simulink model "wlan_phy_rx_pmd"  (Simulink 7.8) (continued)
== system wlan_phy_rx_pmd/FCS & Pkt Buf/Pkt Buf Interface ==
  DSSS data : Inport
  OFDM data : Inport  Port=2
  BRAM IF 64b : Subsystem
  Bus Selector : BusSelector  OutputSignals=byte_valid,byte_index,byte,last_byte
  Bus Selector1 : BusSelector  OutputSignals=byte_valid,byte_index,byte,last_byte
  DSSS Addr Calc : Subsystem
  Logical7 : xbsIndex_r4/Logical  logical_function=OR  inputs=2  en=off  latency=0  precision=Full  arith_type=Unsigned  n_bits=8  bin_pt=2  align_bp=on  dbl_ovrd=off  block_type=logical
      settings: xl_area=[0, 0, 0, 0, 0, 0, 0]  block_version=VER_STRING_GOES_HERE
  MPDU Byte Outputs : Subsystem
  Mux2 : xbsIndex_r4/Mux  inputs=2  en=off  latency=0  precision=Full  arith_type=Unsigned  n_bits=16  bin_pt=14  quantization=Truncate  overflow=Wrap  dbl_ovrd=off  block_type=mux
  Mux3 : xbsIndex_r4/Mux  inputs=2  en=off  latency=0  precision=Full  arith_type=Unsigned  n_bits=16  bin_pt=14  quantization=Truncate  overflow=Wrap  dbl_ovrd=off  block_type=mux
  Mux5 : xbsIndex_r4/Mux  inputs=2  en=off  latency=0  precision=Full  arith_type=Unsigned  n_bits=16  bin_pt=14  quantization=Truncate  overflow=Wrap  dbl_ovrd=off  block_type=mux
  OFDM Addr Calc : Subsystem
  Register{4..5,7..8} (4 alike) : xbsIndex_r4/Register  init=0  rst=off  en=off  dbl_ovrd=off  block_type=register
  wires:
  [DSSS_Byte_Valid] -> Mux3/1
  [DSSS_Byte_Index] -> DSSS Addr Calc/1(R)
  DSSS Addr Calc/1(M) -> Mux5/3
  [DSSS_Byte_Valid] -> Mux5/1
  Mux5/1 -> Register5/1
  [OFDM_Byte_Index] -> OFDM Addr Calc/1(R)
  OFDM Addr Calc/1(M) -> Mux5/2
  Mux3/1 -> Register4/1
  Register4/1 -> BRAM IF 64b/4(Pkt Buf Sel)
  [CUR_RX_BYTE] -> Register7/1
  [CUR_RX_BYTE_VALID] -> Register8/1
  Register7/1 -> BRAM IF 64b/1("byte "), MPDU Byte Outputs/1("Byte ")
  Register8/1 -> BRAM IF 64b/2(byte_valid), MPDU Byte Outputs/2(Byte Valid)
  Register5/1 -> BRAM IF 64b/3(byte_ind), MPDU Byte Outputs/3(Byte Ind)
  Bus Selector1/1 -> [DSSS_Byte_Valid]  "<byte_valid>"
  Bus Selector1/2 -> [DSSS_Byte_Index]  "<byte_index>"
  Bus Selector1/3 -> [DSSS_Byte]  "<byte>"
  Bus Selector1/4 -> [DSSS_Last_Byte]  "<last_byte>"
  Bus Selector/1 -> [OFDM_Byte_Valid]  "<byte_valid>"
  Bus Selector/2 -> [OFDM_Byte_Index]  "<byte_index>"
  Bus Selector/3 -> [OFDM_Byte]  "<byte>"
  Bus Selector/4 -> [OFDM_Last_Byte]  "<last_byte>"
  DSSS data/1 -> Bus Selector1/1
  OFDM data/1 -> Bus Selector/1
  [DSSS_Byte_Valid] -> Mux2/1
  [OFDM_Byte] -> Mux2/2
  [DSSS_Byte] -> Mux2/3
  Mux2/1 -> [CUR_RX_BYTE]
  [DSSS_Byte_Valid] -> Logical7/1
  [OFDM_Byte_Valid] -> Logical7/2
  Logical7/1 -> [CUR_RX_BYTE_VALID]
  [regRx_OFDM_PKT_BUF_SEL] -> Mux3/2
  [regRx_DSSS_PKT_BUF_SEL] -> Mux3/3

== system wlan_phy_rx_pmd/FFT/Reset Gen ==
  note: Extend FFT reset for duration of TVALID + 4 to handle
      | case of pkt ending while new FFT frame is being loaded.
      | Turns out reset inputs for FIFO and FFT do not actually
      | flush internal state - both cores keep respnding to inputs
      | while reset is asserted (very frustrating...)
  FFT Valid In : Inport
  Reset : Inport  Port=2
  Inverter : xbsIndex_r4/Inverter  en=off  latency=0  dbl_ovrd=off  block_type=inv
  Inverter1 : xbsIndex_r4/Inverter  en=off  latency=4  dbl_ovrd=off  block_type=inv
  Logical : xbsIndex_r4/Logical  logical_function=AND  inputs=2  en=off  latency=0  precision=Full  arith_type=Unsigned  n_bits=16  bin_pt=0  align_bp=on  dbl_ovrd=off  block_type=logical
  Logical1 : xbsIndex_r4/Logical  logical_function=OR  inputs=2  en=off  latency=0  precision=Full  arith_type=Unsigned  n_bits=16  bin_pt=0  align_bp=on  dbl_ovrd=off  block_type=logical
  Register2 : xbsIndex_r4/Register  init=1  rst=off  en=off  dbl_ovrd=off  block_type=register
      settings: xl_area=[1 1 0 0 0 0 0]  block_version=VER_STRING_GOES_HERE
  S-R Latch1 : Subsystem
  FFT Reset : Outport
  " Reset" : Outport  Port=2
  wires:
  Inverter1/1 -> S-R Latch1/2(R)
  Reset/1 -> Logical1/1, Logical/2
  Logical/1 -> S-R Latch1/1(S)
  S-R Latch1/1(Q) -> Logical1/2
  Logical1/1 -> " Reset"/1, Inverter/1
  FFT Valid In/1 -> Inverter1/1, Logical/1
  Inverter/1 -> Register2/1
  Register2/1 -> FFT Reset/1

== system wlan_phy_rx_pmd/FFT/Sym Count ==
  data_in_tvalid : Inport
  pkt_done : Inport  Port=2
  data_out_tlast : Inport  Port=3
  Logical : xbsIndex_r4/Logical  logical_function=AND  inputs=2  en=off  latency=0  precision=Full  arith_type=Unsigned  n_bits=16  bin_pt=0  align_bp=on  dbl_ovrd=off  block_type=logical
  S-R Latch1 : Subsystem
  Symbol Counter : xbsIndex_r4/Counter  cnt_type=Free Running  cnt_to=Inf  operation=Up  start_count=0  cnt_by_val=1  arith_type=Unsigned  n_bits=ceil(log2(MAX_NUM_SYMS))  bin_pt=0  load_pin=off  rst=on  en=on  explicit_period=off  period=1  dbl_ovrd=off  use_behavioral_HDL=off  implementation=Fabric  block_type=counter
  Sym Ind : Outport
  wires:
  data_out_tlast/1 -> Logical/1
  Logical/1 -> Symbol Counter/2
  S-R Latch1/1(Q) -> Logical/2
  data_in_tvalid/1 -> S-R Latch1/1(S)
  Symbol Counter/1 -> Sym Ind/1
  pkt_done/1 -> S-R Latch1/2(R), Symbol Counter/1

== system wlan_phy_rx_pmd/Inputs/RF A RSSI Sum ==
  RSSI : Inport
  Down Sample6 : xbsIndex_r4/Down Sample  sample_ratio=16  sample_phase="Last Value of Frame  (most efficient)"  en=off  latency=1  dbl_ovrd=off  block_type=dsamp
      settings: block_version=VER_STRING_GOES_HERE
  RSSI DET Debug Out : Subsystem
  Running Sum : Subsystem
  Sum : Outport
  wires:
  [RX_GLOBAL_RESET] -> Down Sample6/1
  RSSI/1 -> Running Sum/1(RSSI), RSSI DET Debug Out/2(Raw RSSI)
  Down Sample6/1 -> Running Sum/2(reset)
  Running Sum/1(avg) -> Sum/1, RSSI DET Debug Out/1(Avg RSSI)

== system wlan_phy_rx_pmd/Inputs/RF B RSSI Sum ==
  RSSI : Inport
  Down Sample6 : xbsIndex_r4/Down Sample  sample_ratio=16  sample_phase="Last Value of Frame  (most efficient)"  en=off  latency=1  dbl_ovrd=off  block_type=dsamp
      settings: block_version=VER_STRING_GOES_HERE
  Running Sum : Subsystem
  Sum : Outport
  wires:
  Running Sum/1(avg) -> Sum/1
  Down Sample6/1 -> Running Sum/2(reset)
  RSSI/1 -> Running Sum/1(RSSI)
  [RX_GLOBAL_RESET] -> Down Sample6/1

== system wlan_phy_rx_pmd/Inputs/RSSI Clock Gen ==
  1LSB+3 : xbsIndex_r4/Slice  nbits=1  boolean_output=off  mode=Lower Bit Location + Width  bit1=0  base1=MSB of Input  bit0=3  base0=LSB of Input  dbl_ovrd=off  block_type=slice
  RSSI Clock Generator : xbsIndex_r4/Counter  cnt_type=Free Running  cnt_to=Inf  operation=Up  start_count=0  cnt_by_val=1  arith_type=Unsigned  n_bits=4  bin_pt=0  load_pin=off  rst=off  en=off  explicit_period=on  period=1  dbl_ovrd=off  use_behavioral_HDL=off  implementation=Fabric  use_rpm=off  block_type=counter
      settings: block_version=VER_STRING_GOES_HERE
  Clk : Outport
  wires:
  RSSI Clock Generator/1 -> 1LSB+3/1
  1LSB+3/1 -> Clk/1

== system wlan_phy_rx_pmd/MAC Outputs/S-R Latch1 ==
  S : Inport
  R : Inport  Port=2
  Constant1 : xbsIndex_r4/Constant  const=1  gui_display_data_type=Fixed-point  arith_type=Boolean  n_bits=1  bin_pt=0  preci_type=Single  exp_width=8  frac_width=24  explicit_period=off  period=1  equ=P=C  opselect=C  inp2=PCIN>>17  opr=+  inp1=P  carry=CIN  dbl_ovrd=off  block_type=constant
      settings: block_version=VER_STRING_GOES_HERE
  Convert1 : xbsIndex_r4/Convert  gui_display_data_type=Boolean  arith_type=Boolean  n_bits=1  bin_pt=0  float_type=Single  exp_bits=8  fraction_bits=24  quantization=Truncate  overflow=Wrap  en=off  latency=0  dbl_ovrd=off  pipeline=off  block_type=convert
  Convert2 : xbsIndex_r4/Convert  gui_display_data_type=Boolean  arith_type=Boolean  n_bits=1  bin_pt=0  float_type=Single  exp_bits=8  fraction_bits=24  quantization=Truncate  overflow=Wrap  en=off  latency=0  dbl_ovrd=off  pipeline=off  block_type=convert
  Register2 : xbsIndex_r4/Register  init=0  rst=on  en=on  dbl_ovrd=off  block_type=register
      settings: xl_area=[1 1 0 0 0 0 0]  block_version=VER_STRING_GOES_HERE
  Q : Outport
  wires:
  Convert2/1 -> Register2/3
  Convert1/1 -> Register2/2
  Constant1/1 -> Register2/1
  R/1 -> Convert1/1
  Register2/1 -> Q/1
  S/1 -> Convert2/1

== system wlan_phy_rx_pmd/Registers/2x  ==
  d : Inport
  Concat2 : xbsIndex_r4/Concat  num_inputs=2  dbl_ovrd=off  block_type=concat
      settings: block_version=8.2.02
  Constant2 : xbsIndex_r4/Constant  const=0  gui_display_data_type=Fixed-point  arith_type=Unsigned  n_bits=1  bin_pt=0  preci_type=Single  exp_width=8  frac_width=24  explicit_period=on  period=1  equ=P=C  opselect=C  inp2=PCIN>>17  opr=+  inp1=P  carry=CIN  dbl_ovrd=off  block_type=constant
  2d : Outport
  wires:
  Concat2/1 -> 2d/1
  d/1 -> Concat2/1
  Constant2/1 -> Concat2/2

== system wlan_phy_rx_pmd/Registers/Config Bits ==
  32b : Inport
  "b[0]   " : xbsIndex_r4/Slice  nbits=1  boolean_output=on  mode=Lower Bit Location + Width  bit1=0  base1=MSB of Input  bit0=0  base0=LSB of Input  dbl_ovrd=off  block_type=slice
      settings: block_version=VER_STRING_GOES_HERE
  b[10] : xbsIndex_r4/Slice  nbits=1  boolean_output=on  mode=Lower Bit Location + Width  bit1=0  base1=MSB of Input  bit0=10  base0=LSB of Input  dbl_ovrd=off  block_type=slice
      settings: block_version=VER_STRING_GOES_HERE
  b[11] : xbsIndex_r4/Slice  nbits=1  boolean_output=on  mode=Lower Bit Location + Width  bit1=0  base1=MSB of Input  bit0=11  base0=LSB of Input  dbl_ovrd=off  block_type=slice
      settings: block_version=VER_STRING_GOES_HERE
  "b[1]  " : xbsIndex_r4/Slice  nbits=1  boolean_output=on  mode=Lower Bit Location + Width  bit1=0  base1=MSB of Input  bit0=1  base0=LSB of Input  dbl_ovrd=off  block_type=slice
      settings: block_version=VER_STRING_GOES_HERE
  b[2] : xbsIndex_r4/Slice  nbits=1  boolean_output=off  mode=Lower Bit Location + Width  bit1=0  base1=MSB of Input  bit0=2  base0=LSB of Input  dbl_ovrd=off  block_type=slice
      settings: block_version=VER_STRING_GOES_HERE
  b[3] : xbsIndex_r4/Slice  nbits=1  boolean_output=off  mode=Lower Bit Location + Width  bit1=0  base1=MSB of Input  bit0=3  base0=LSB of Input  dbl_ovrd=off  block_type=slice
      settings: block_version=VER_STRING_GOES_HERE
  b[4] : xbsIndex_r4/Slice  nbits=1  boolean_output=off  mode=Lower Bit Location + Width  bit1=0  base1=MSB of Input  bit0=4  base0=LSB of Input  dbl_ovrd=off  block_type=slice
      settings: block_version=VER_STRING_GOES_HERE
  b[5] : xbsIndex_r4/Slice  nbits=1  boolean_output=on  mode=Lower Bit Location + Width  bit1=0  base1=MSB of Input  bit0=5  base0=LSB of Input  dbl_ovrd=off  block_type=slice
      settings: block_version=VER_STRING_GOES_HERE
  b[6] : xbsIndex_r4/Slice  nbits=1  boolean_output=on  mode=Lower Bit Location + Width  bit1=0  base1=MSB of Input  bit0=6  base0=LSB of Input  dbl_ovrd=off  block_type=slice
      settings: block_version=VER_STRING_GOES_HERE
  b[7] : xbsIndex_r4/Slice  nbits=1  boolean_output=on  mode=Lower Bit Location + Width  bit1=0  base1=MSB of Input  bit0=7  base0=LSB of Input  dbl_ovrd=off  block_type=slice
      settings: block_version=VER_STRING_GOES_HERE
  b[8] : xbsIndex_r4/Slice  nbits=1  boolean_output=on  mode=Lower Bit Location + Width  bit1=0  base1=MSB of Input  bit0=8  base0=LSB of Input  dbl_ovrd=off  block_type=slice
      settings: block_version=VER_STRING_GOES_HERE
  b[9] : xbsIndex_r4/Slice  nbits=1  boolean_output=on  mode=Lower Bit Location + Width  bit1=0  base1=MSB of Input  bit0=9  base0=LSB of Input  dbl_ovrd=off  block_type=slice
      settings: block_version=VER_STRING_GOES_HERE
  tag scope: REG_USE_RX_SIGS_INVALID_INPUT=global, regRx_BYPASS_CFO_EST=global, regRx_DSSS_RX_EN=global, regRx_DSSS_RX_PKT_DET_OUT_EN=global, regRx_PKT_BUF_BYTE_ORDER_SWAP=global, regRx_PKT_BUF_H_EST_ORDER_SWAP=global, regRx_Record_Chan_Est_Pkt_Buf=global, regRx_noSwitching_AntSel=global, regRx_pktDetEn_Ext=global, regRx_pktDetEn_RFA=global, regRx_pktDetEn_RFB=global, regRx_switchingDiv_En=global
  wires:
  32b/1 -> "b[1]  "/1, b[2]/1, b[3]/1, b[4]/1, b[5]/1, b[6]/1, b[7]/1, b[8]/1, b[9]/1, b[10]/1, b[11]/1, "b[0]   "/1
  "b[0]   "/1 -> [regRx_DSSS_RX_EN]
  "b[1]  "/1 -> [REG_USE_RX_SIGS_INVALID_INPUT]
  b[2]/1 -> [regRx_PKT_BUF_BYTE_ORDER_SWAP]
  b[3]/1 -> [regRx_PKT_BUF_H_EST_ORDER_SWAP]
  b[4]/1 -> [regRx_DSSS_RX_PKT_DET_OUT_EN]
  b[5]/1 -> [regRx_BYPASS_CFO_EST]
  b[6]/1 -> [regRx_Record_Chan_Est_Pkt_Buf]
  b[7]/1 -> [regRx_switchingDiv_En]
  b[8]/1 -> [regRx_noSwitching_AntSel]
  b[9]/1 -> [regRx_pktDetEn_RFA]
  b[10]/1 -> [regRx_pktDetEn_RFB]
  b[11]/1 -> [regRx_pktDetEn_Ext]

== system wlan_phy_rx_pmd/Registers/Sim Reset ==
  Add : Sum
  Disregard Subsystem : xbsIndex_r4/Disregard Subsystem  Tag=discardX  block_type=disregard
  Pulse Generator : DiscretePulseGenerator  Period=1e6  PulseWidth=2  PhaseDelay=1.25e4
  Pulse Generator1 : DiscretePulseGenerator  Period=1e6  PulseWidth=2  PhaseDelay=2.5e4
  sim_only_reset : xbsIndex_r4/Gateway In  gui_display_data_type=Fixed-point  arith_type=Unsigned  n_bits=1  bin_pt=0  preci_type=Single  exp_width=8  frac_width=24  quantization="Round  (unbiased: +/- Inf)"  overflow=Saturate  period=1  dbl_ovrd=off  timing_constraint=None  locs_specified=off  LOCs={}  UseAsADC=off  ADCChannel='1'  inherit_from_input=off  hdl_port=on  block_type=gatewayin
  Out1 : Outport
  wires:
  sim_only_reset/1 -> Out1/1
  Pulse Generator/1 -> Add/1
  Pulse Generator1/1 -> Add/2
  Add/1 -> sim_only_reset/1

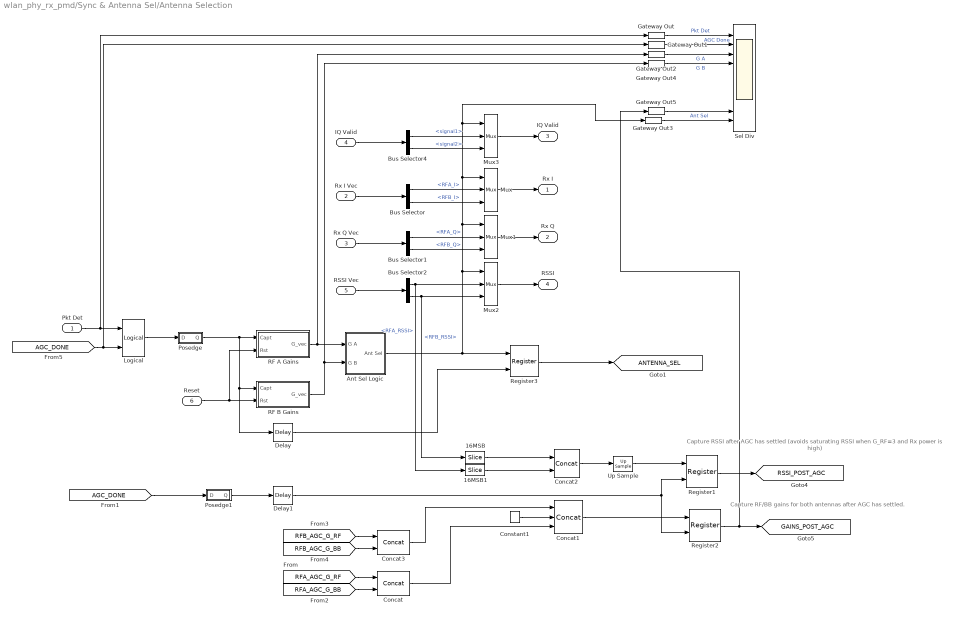
== system wlan_phy_rx_pmd/Sync & Antenna Sel/Antenna Selection ==
  note: Capture RF/BB gains for both antennas
      | after AGC has settled.
  note: Capture RSSI after AGC has settled
      | (avoids saturating RSSI when G_RF=3
      | and Rx power is high)
  Pkt Det : Inport
  Rx I Vec : Inport  Port=2
  Rx Q Vec : Inport  Port=3
  IQ Valid : Inport  Port=4
  RSSI Vec : Inport  Port=5
  Reset : Inport  Port=6
  16MSB : xbsIndex_r4/Slice  nbits=16  boolean_output=off  mode=Upper Bit Location + Width  bit1=0  base1=MSB of Input  bit0=0  base0=LSB of Input  dbl_ovrd=off  block_type=slice
  16MSB1 : xbsIndex_r4/Slice  nbits=16  boolean_output=off  mode=Upper Bit Location + Width  bit1=0  base1=MSB of Input  bit0=0  base0=LSB of Input  dbl_ovrd=off  block_type=slice
  Ant Sel Logic : Subsystem
  Bus Selector : BusSelector  OutputSignals=RFA_I,RFB_I
  Bus Selector1 : BusSelector  OutputSignals=RFA_Q,RFB_Q
  Bus Selector2 : BusSelector  OutputSignals=RFA_RSSI,RFB_RSSI
  Bus Selector4 : BusSelector  OutputSignals=signal1,signal2
  Concat : xbsIndex_r4/Concat  num_inputs=2  dbl_ovrd=off  block_type=concat
  Concat1 : xbsIndex_r4/Concat  num_inputs=3  dbl_ovrd=off  block_type=concat
  Concat2 : xbsIndex_r4/Concat  num_inputs=2  dbl_ovrd=off  block_type=concat
  Concat3 : xbsIndex_r4/Concat  num_inputs=2  dbl_ovrd=off  block_type=concat
  Constant1 : xbsIndex_r4/Constant  const=0  gui_display_data_type=Fixed-point  arith_type=Unsigned  n_bits=1  bin_pt=0  preci_type=Single  exp_width=8  frac_width=24  explicit_period=off  period=1  equ=P=C  opselect=C  inp2=PCIN>>17  opr=+  inp1=P  carry=CIN  dbl_ovrd=off  block_type=constant
      settings: block_version=VER_STRING_GOES_HERE
  Delay : xbsIndex_r4/Delay  rst=off  infoeditControl=Selection of Reset will increase slice count due to use of real FFs and instead of SRLs  en=off  latency=1  dbl_ovrd=off  reg_retiming=off  block_type=delay
  Delay1 : xbsIndex_r4/Delay  rst=off  infoeditControl=Selection of Reset will increase slice count due to use of real FFs and instead of SRLs  en=off  latency=4  dbl_ovrd=off  reg_retiming=off  block_type=delay
  Gateway Out{,1..5} (6 alike) : xbsIndex_r4/Gateway Out  inherit_from_input=off  hdl_port=off  timing_constraint=None  locs_specified=off  LOCs={}  UseAsDAC=off  DACChannel='1'  block_type=gatewayout
  Logical : xbsIndex_r4/Logical  logical_function=AND  inputs=2  en=off  latency=0  precision=Full  arith_type=Unsigned  n_bits=16  bin_pt=0  align_bp=on  dbl_ovrd=off  block_type=logical
  Mux{,1..3} (4 alike) : xbsIndex_r4/Mux  inputs=2  en=off  latency=0  precision=Full  arith_type=Unsigned  n_bits=16  bin_pt=14  quantization=Truncate  overflow=Wrap  dbl_ovrd=off  block_type=mux
  Posedge : Subsystem
  Posedge1 : Subsystem
  RF A Gains : Subsystem
  RF B Gains : Subsystem
  Register1 : xbsIndex_r4/Register  init=0  rst=off  en=on  dbl_ovrd=off  block_type=register
  Register2 : xbsIndex_r4/Register  init=0  rst=off  en=on  dbl_ovrd=off  block_type=register
  Register3 : xbsIndex_r4/Register  init=0  rst=off  en=on  dbl_ovrd=off  block_type=register
      settings: xl_area=[1 1 0 0 0 0 0]  block_version=VER_STRING_GOES_HERE
  Sel Div : Scope  NumInputPorts=10
      settings: TimeRange=16708  YMin=0~-1~-1~0~-1~-1~-1~-1~-1~-1  YMax=1~1~1~1~1~1~1~1~1~1  SaveName=ScopeData42
  Up Sample : xbsIndex_r4/Up Sample  sample_ratio=16  copy_samples=on  en=off  latency=0  dbl_ovrd=off  block_type=usamp
  Rx I : Outport
  " Rx Q" : Outport  Port=2
  " IQ Valid" : Outport  Port=3
  RSSI : Outport  Port=4
  tag scope: ANTENNA_SEL=global, GAINS_POST_AGC=global, RSSI_POST_AGC=global
  wires:
  RF A Gains/1(G_vec) -> Ant Sel Logic/1(G A), Gateway Out2/1
  RF B Gains/1(G_vec) -> Ant Sel Logic/2(G B), Gateway Out4/1
  Rx I Vec/1 -> Bus Selector/1
  Bus Selector/1 -> Mux/2  "<RFA_I>"
  Bus Selector/2 -> Mux/3  "<RFB_I>"
  Bus Selector1/1 -> Mux1/2  "<RFA_Q>"
  Bus Selector1/2 -> Mux1/3  "<RFB_Q>"
  Rx Q Vec/1 -> Bus Selector1/1
  Bus Selector2/1 -> Mux2/2, 16MSB1/1  "<RFA_RSSI>"
  Bus Selector2/2 -> Mux2/3, 16MSB/1  "<RFB_RSSI>"
  RSSI Vec/1 -> Bus Selector2/1
  Mux/1 -> Rx I/1
  Mux1/1 -> " Rx Q"/1
  Reset/1 -> RF B Gains/2(Rst), RF A Gains/2(Rst)
  Logical/1 -> Posedge/1(D)
  Ant Sel Logic/1(Ant Sel) -> Mux1/1, Mux/1, Mux3/1, Gateway Out3/1, Mux2/1, Register3/1
  Mux2/1 -> RSSI/1
  Pkt Det/1 -> Logical/1, Gateway Out/1
  [AGC_DONE] -> Logical/2, Gateway Out1/1
  Posedge/1(Q) -> RF A Gains/1(Capt), RF B Gains/1(Capt), Delay/1
  Concat1/1 -> Register2/1
  Register2/1 -> [GAINS_POST_AGC], Gateway Out5/1
  Register1/1 -> [RSSI_POST_AGC]
  Constant1/1 -> Concat1/2
  Delay/1 -> Register3/2
  Register3/1 -> [ANTENNA_SEL]
  Concat2/1 -> Up Sample/1
  Up Sample/1 -> Register1/1
  IQ Valid/1 -> Bus Selector4/1
  Bus Selector4/1 -> Mux3/2  "<signal1>"
  Bus Selector4/2 -> Mux3/3  "<signal2>"
  Mux3/1 -> " IQ Valid"/1
  16MSB/1 -> Concat2/1
  16MSB1/1 -> Concat2/2
  Gateway Out2/1 -> Sel Div/3  "G A"
  Gateway Out1/1 -> Sel Div/2  "AGC Done"
  Gateway Out/1 -> Sel Div/1  "Pkt Det"
  Gateway Out3/1 -> Sel Div/10  "Ant Sel"
  Gateway Out4/1 -> Sel Div/4  "G B"
  Gateway Out5/1 -> Sel Div/9
  Delay1/1 -> Register1/2, Register2/2
  [AGC_DONE] -> Posedge1/1(D)
  Posedge1/1(Q) -> Delay1/1
  [RFA_AGC_G_RF] -> Concat/1
  [RFA_AGC_G_BB] -> Concat/2
  [RFB_AGC_G_BB] -> Concat3/2
  [RFB_AGC_G_RF] -> Concat3/1
  Concat3/1 -> Concat1/1
  Concat/1 -> Concat1/3

== system wlan_phy_rx_pmd/Sync & Antenna Sel/Debug  Outputs ==
  note: Pulse stretch the timeout signal
      | so it's visible on debug outputs
      | and in down-sampled CS ILA
  Pkt Det : Inport
  LTS : Inport  Port=2
  LTS Timeout : Inport  Port=3
  Delay : xbsIndex_r4/Delay  rst=off  infoeditControl=Selection of Reset will increase slice count due to use of real FFs and instead of SRLs  en=off  latency=16  dbl_ovrd=off  reg_retiming=off  block_type=delay
  Delay1 : xbsIndex_r4/Delay  rst=off  infoeditControl=Selection of Reset will increase slice count due to use of real FFs and instead of SRLs  en=off  latency=16  dbl_ovrd=off  reg_retiming=off  block_type=delay
  LTS_SYNC : xbsIndex_r4/Gateway Out  inherit_from_input=off  hdl_port=on  timing_constraint=None  locs_specified=off  LOCs={}  UseAsDAC=off  DACChannel='1'  block_type=gatewayout
  Register{,1..3,8..9} (6 alike) : xbsIndex_r4/Register  init=0  rst=off  en=off  dbl_ovrd=off  block_type=register
  S-R Latch : Subsystem
  S-R Latch1 : Subsystem
  Slice2 : xbsIndex_r4/Slice  nbits=1  boolean_output=on  mode=Upper Bit Location + Width  bit1=0  base1=MSB of Input  bit0=0  base0=LSB of Input  dbl_ovrd=off  block_type=slice
  dbg_lts_timeout : xbsIndex_r4/Gateway Out  inherit_from_input=off  hdl_port=on  timing_constraint=None  locs_specified=off  LOCs={}  UseAsDAC=off  DACChannel='1'  block_type=gatewayout
  dbg_pkt_det : xbsIndex_r4/Gateway Out  inherit_from_input=off  hdl_port=on  timing_constraint=None  locs_specified=off  LOCs={}  UseAsDAC=off  DACChannel='1'  block_type=gatewayout
  tag scope: CS_LTS_Timeout=global, CS_Pktdet=global
  wires:
  Register/1 -> Register1/1
  Register1/1 -> dbg_pkt_det/1
  S-R Latch/1(Q) -> Delay/1, Register8/1
  Delay/1 -> S-R Latch/2(R)
  Register8/1 -> Register9/1
  Register9/1 -> LTS_SYNC/1
  Slice2/1 -> S-R Latch/1(S)
  Pkt Det/1 -> [CS_Pktdet], Register/1
  LTS Timeout/1 -> S-R Latch1/1(S)
  Register2/1 -> Register3/1
  Register3/1 -> dbg_lts_timeout/1
  Delay1/1 -> S-R Latch1/2(R)
  S-R Latch1/1(Q) -> Register2/1, [CS_LTS_Timeout], Delay1/1
  LTS/1 -> Slice2/1

== system wlan_phy_rx_pmd/Sync & Antenna Sel/LTS Correlation ==
  rx_I : Inport
  rx_Q : Inport  Port=2
  rx_IQ_valid : Inport  Port=3
  RSSI : Inport  Port=4
  En : Inport  Port=5
  Reset : Inport  Port=6
  Bus Creator : BusCreator  Inputs=2
  Bus Creator1 : BusCreator  Inputs=2
  Concat : xbsIndex_r4/Concat  num_inputs=2  dbl_ovrd=off  block_type=concat
  Correlator : Subsystem
  Gateway Out{1..4,6..10} (9 alike) : xbsIndex_r4/Gateway Out  inherit_from_input=off  hdl_port=off  timing_constraint=None  locs_specified=off  LOCs={}  UseAsDAC=off  DACChannel='1'  block_type=gatewayout
  Inverter : xbsIndex_r4/Inverter  en=off  latency=0  dbl_ovrd=off  block_type=inv
      settings: xl_area=[0 0 0 0 0 0 0]  block_version=VER_STRING_GOES_HERE
  Inverter1 : xbsIndex_r4/Inverter  en=off  latency=0  dbl_ovrd=off  block_type=inv
      settings: xl_area=[0 0 0 0 0 0 0]  block_version=VER_STRING_GOES_HERE
  LTS Corr : Scope  NumInputPorts=8
      settings: TimeRange=7000  YMin=-1~-1~0~-1~-1~0~0~-1  YMax=1~1~15000~1~1~1~1~1  SaveName=ScopeData2
  LTS Corr Timeout : Subsystem
  Logical1 : xbsIndex_r4/Logical  logical_function=AND  inputs=2  en=off  latency=0  precision=Full  arith_type=Unsigned  n_bits=16  bin_pt=0  align_bp=on  dbl_ovrd=off  block_type=logical
  Logical2 : xbsIndex_r4/Logical  logical_function=AND  inputs=3  en=off  latency=0  precision=Full  arith_type=Unsigned  n_bits=16  bin_pt=0  align_bp=on  dbl_ovrd=off  block_type=logical
  Logical3 : xbsIndex_r4/Logical  logical_function=AND  inputs=2  en=off  latency=1  precision=Full  arith_type=Unsigned  n_bits=16  bin_pt=0  align_bp=on  dbl_ovrd=off  block_type=logical
  Posedge3 : Subsystem
  Relational2 : xbsIndex_r4/Relational  mode=a>b  en=off  latency=0  dbl_ovrd=off  block_type=relational
      settings: xl_area=[10 0 0 19 0 0 0]  block_version=VER_STRING_GOES_HERE
  S-R Latch : Subsystem
  S-R Latch1 : Subsystem
  Slice : xbsIndex_r4/Slice  nbits=1  boolean_output=off  mode=Upper Bit Location + Width  bit1=0  base1=MSB of Input  bit0=0  base0=LSB of Input  dbl_ovrd=off  block_type=slice
      settings: infoedit="Extracts a given range of bits from each input sample and presents it at the output.  The output type is ordinarily unsigned with binary point at zero, but can be Boolean when the slice is one bit wide.<P><P>Hardware notes: In hardware this block costs nothing."  block_version=10.1.3
  Slice1 : xbsIndex_r4/Slice  nbits=1  boolean_output=off  mode=Upper Bit Location + Width  bit1=0  base1=MSB of Input  bit0=0  base0=LSB of Input  dbl_ovrd=off  block_type=slice
      settings: infoedit="Extracts a given range of bits from each input sample and presents it at the output.  The output type is ordinarily unsigned with binary point at zero, but can be Boolean when the slice is one bit wide.<P><P>Hardware notes: In hardware this block costs nothing."  block_version=10.1.3
  Threshold Select : Subsystem
  Det : Outport
  Timeout : Outport  Port=2
  tag scope: CS_LTS_Det_1=global, CS_LTS_Det_2=global
  wires:
  Gateway Out2/1 -> LTS Corr/2
  Gateway Out4/1 -> LTS Corr/1
  Logical1/1 -> LTS Corr Timeout/3(LTS 1), Concat/2, [CS_LTS_Det_1], Gateway Out8/1
  Inverter/1 -> Logical1/2
  Reset/1 -> S-R Latch/2(R), S-R Latch1/2(R), Gateway Out10/1
  Logical2/1 -> S-R Latch1/1(S), LTS Corr Timeout/4(LTS 2), Concat/1, [CS_LTS_Det_2], Gateway Out9/1
  S-R Latch/1(Q) -> Inverter/1, Logical2/3
  Gateway Out1/1 -> LTS Corr/3
  Gateway Out3/1 -> LTS Corr/4
  rx_I/1 -> Slice/1, Gateway Out4/1
  rx_Q/1 -> Slice1/1, Gateway Out2/1
  Correlator/1(R) -> Relational2/1, Gateway Out1/1
  Gateway Out7/1 -> LTS Corr/7
  Posedge3/1(Q) -> Logical1/1, Logical2/2, S-R Latch/1(S), Gateway Out3/1
  Logical3/1 -> Posedge3/1(D)
  Relational2/1 -> Logical3/2
  En/1 -> LTS Corr Timeout/1(Pkt Det), Logical3/1, Gateway Out6/1, Threshold Select/1(Pkt Det)
  S-R Latch1/1(Q) -> Inverter1/1
  Inverter1/1 -> Logical2/1
  LTS Corr Timeout/1(Timeout) -> Timeout/1, Gateway Out7/1
  Slice/1 -> Correlator/1(Rx I)
  Slice1/1 -> Correlator/2(Rx Q)
  rx_IQ_valid/1 -> LTS Corr Timeout/2(IQ Valid), Correlator/3(rx_IQ_valid)
  Bus Creator/1 -> LTS Corr/5
  Bus Creator1/1 -> LTS Corr/6
  Gateway Out8/1 -> Bus Creator/1
  Gateway Out9/1 -> Bus Creator/2
  Gateway Out6/1 -> Bus Creator1/1
  Gateway Out10/1 -> Bus Creator1/2
  Threshold Select/1(Corr Thresh) -> Relational2/2
  RSSI/1 -> Threshold Select/2(RSSI)
  Concat/1 -> Det/1

== system wlan_phy_rx_pmd/Sync & Antenna Sel/PHY CCA ==
  LTS Sync : Inport
  LTS Timeout : Inport  Port=2
  IQ Valid : Inport  Port=3
  Pkt Det : Inport  Port=4
  Avg RSSI Vec : Inport  Port=5
  Reset : Inport  Port=6
  Ant Sel Logic : Subsystem
  Bus Selector1 : BusSelector  OutputSignals=RFA_RSSI,RFB_RSSI
  Gateway Out{1..2,4..5,8} (5 alike) : xbsIndex_r4/Gateway Out  inherit_from_input=off  hdl_port=off  timing_constraint=None  locs_specified=off  LOCs={}  UseAsDAC=off  DACChannel='1'  block_type=gatewayout
  Logical1 : xbsIndex_r4/Logical  logical_function=OR  inputs=2  en=off  latency=0  precision=Full  arith_type=Unsigned  n_bits=16  bin_pt=0  align_bp=on  dbl_ovrd=off  block_type=logical
  Logical2 : xbsIndex_r4/Logical  logical_function=OR  inputs=3  en=off  latency=0  precision=Full  arith_type=Unsigned  n_bits=16  bin_pt=0  align_bp=on  dbl_ovrd=off  block_type=logical
  Logical3 : xbsIndex_r4/Logical  logical_function=AND  inputs=2  en=off  latency=0  precision=Full  arith_type=Unsigned  n_bits=16  bin_pt=0  align_bp=on  dbl_ovrd=off  block_type=logical
  PHY CCA : Scope  NumInputPorts=7
      settings: TimeRange=10000  YMin=-100~-100~-100~-70~0~0~-0.25  YMax=100~75~75~80~1~60000~0.3  SaveName=ScopeData31
  Rate*Length BUSY : Subsystem
  Relational : xbsIndex_r4/Relational  mode=a>b  en=off  latency=0  dbl_ovrd=off  block_type=relational
  Relational1 : xbsIndex_r4/Relational  mode=a>b  en=off  latency=0  dbl_ovrd=off  block_type=relational
  S-R Latch2 : Subsystem
  Slice2 : xbsIndex_r4/Slice  nbits=1  boolean_output=on  mode=Upper Bit Location + Width  bit1=0  base1=MSB of Input  bit0=0  base0=LSB of Input  dbl_ovrd=off  block_type=slice
  tag scope: RX_CCA_BUSY=global
  wires:
  [regRx_RSSI_CS_THRESH] -> Relational/2, Relational1/2
  Bus Selector1/1 -> Relational/1, Gateway Out4/1  "<RFA_RSSI>"
  Logical2/1 -> Gateway Out8/1, [RX_CCA_BUSY]  "CCA_BUSY"
  Pkt Det/1 -> S-R Latch2/1(S)
  Logical1/1 -> S-R Latch2/2(R)
  Reset/1 -> Logical1/1
  LTS Timeout/1 -> Logical1/2
  S-R Latch2/1(Q) -> Logical2/3, Gateway Out5/1, Logical3/2
  IQ Valid/1 -> Rate*Length BUSY/1(IQ Valid)
  Logical3/1 -> Rate*Length BUSY/2(Start), Gateway Out1/1
  Rate*Length BUSY/1(PHY Rx Active) -> Logical2/1, Gateway Out2/1
  Gateway Out4/1 -> PHY CCA/1
  Gateway Out1/1 -> PHY CCA/2
  Gateway Out2/1 -> PHY CCA/3
  Gateway Out5/1 -> PHY CCA/5
  Gateway Out8/1 -> PHY CCA/6
  LTS Sync/1 -> Slice2/1
  Avg RSSI Vec/1 -> Bus Selector1/1
  Slice2/1 -> Logical3/1
  Relational/1 -> Ant Sel Logic/1(Det A)
  Relational1/1 -> Ant Sel Logic/2(Det B)
  Bus Selector1/2 -> Relational1/1  "<RFB_RSSI>"
  Ant Sel Logic/1(Pkt Det) -> Logical2/2
